simulink model "All_64_Coils"  (R2021b)
settings: Solver=FixedStepAuto  StartTime=-3000e-6  StopTime=3000e-6  FixedStep=25e-6  RelTol=1e-3
(part 1 of 70)
settings Model: Creator=teja  LastModifiedBy=teja

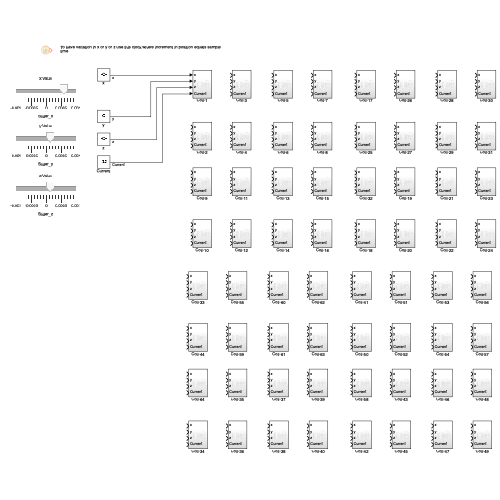
[thumbnail: Simulink's stored preview of the top-level diagram]
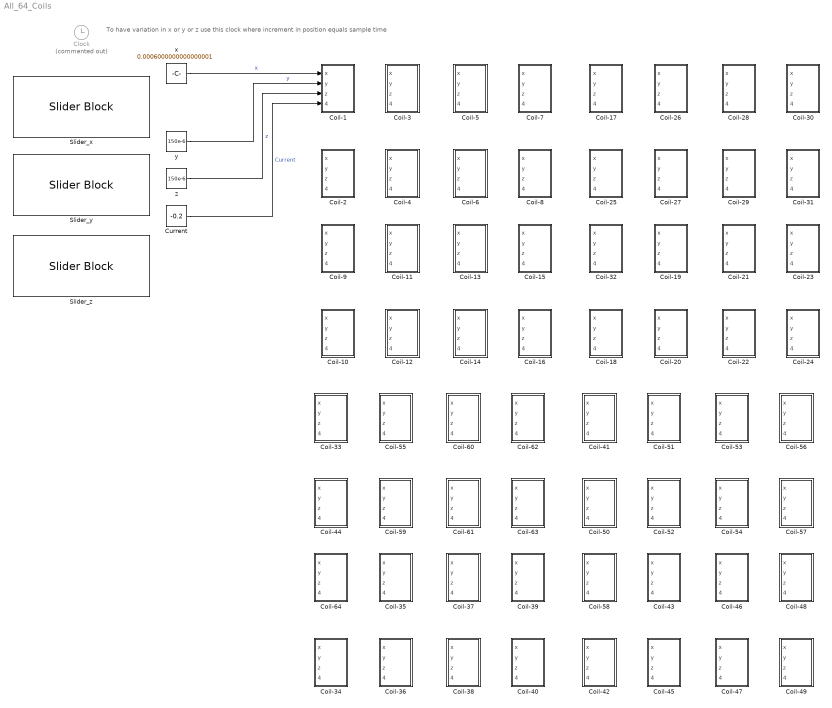
== system All_64_Coils ==
  note: To have variation in x or y or z use this clock where increment in position equals sample time
  Clock : Clock  Commented=on
  Coil-1 : Subsystem
  Coil-10 : Subsystem
  Coil-11 : Subsystem
  Coil-12 : Subsystem
  Coil-13 : Subsystem
  Coil-14 : Subsystem
  Coil-15 : Subsystem
  Coil-16 : Subsystem
  Coil-17 : Subsystem
  Coil-18 : Subsystem
  Coil-19 : Subsystem
  Coil-2 : Subsystem
  Coil-20 : Subsystem
  Coil-21 : Subsystem
  Coil-22 : Subsystem
  Coil-23 : Subsystem
  Coil-24 : Subsystem
  Coil-25 : Subsystem
  Coil-26 : Subsystem
  Coil-27 : Subsystem
  Coil-28 : Subsystem
  Coil-29 : Subsystem
  Coil-3 : Subsystem
  Coil-30 : Subsystem
  Coil-31 : Subsystem
  Coil-32 : Subsystem
  Coil-33 : Subsystem
  Coil-34 : Subsystem
  Coil-35 : Subsystem
  Coil-36 : Subsystem
  Coil-37 : Subsystem
  Coil-38 : Subsystem
  Coil-39 : Subsystem
  Coil-4 : Subsystem
  Coil-40 : Subsystem
  Coil-41 : Subsystem
  Coil-42 : Subsystem
  Coil-43 : Subsystem
  Coil-44 : Subsystem
  Coil-45 : Subsystem
  Coil-46 : Subsystem
  Coil-47 : Subsystem
  Coil-48 : Subsystem
  Coil-49 : Subsystem
  Coil-5 : Subsystem
  Coil-50 : Subsystem
  Coil-51 : Subsystem
  Coil-52 : Subsystem
  Coil-53 : Subsystem
  Coil-54 : Subsystem
  Coil-55 : Subsystem
  Coil-56 : Subsystem
  Coil-57 : Subsystem
  Coil-58 : Subsystem
  Coil-59 : Subsystem
  Coil-6 : Subsystem
  Coil-60 : Subsystem
  Coil-61 : Subsystem
  Coil-62 : Subsystem
  Coil-63 : Subsystem
  Coil-64 : Subsystem
  Coil-7 : Subsystem
  Coil-8 : Subsystem
  Coil-9 : Subsystem
  Current : Constant  Value=-0.2
  Slider_x : SliderBlock  ScaleMin=-1000e-6  ScaleMax=1000e-6
  Slider_y : SliderBlock  ScaleMin=-1e-3  ScaleMax=1e-3
  Slider_z : SliderBlock  ScaleMin=-1000e-6  ScaleMax=1000e-6
  x : Constant  Value=0.0006000000000000001
  y : Constant  Value=150e-6
  z : Constant  Value=150e-6
  wires:
  x/1 -> Coil-1/1(x)  "x"
  y/1 -> Coil-1/2(y)  "y"
  z/1 -> Coil-1/3(z)  "z"
  Current/1 -> Coil-1/4(Current)  "Current"

== system All_64_Coils/Coil-1 ==
  x : Inport
  y : Inport  Port=2
  z : Inport  Port=3
  Current : Inport  Port=4
  Add{,1..4} (5 alike) : Sum
  Display{,1..3} (4 alike) : Display
  Inner_5_coils : Subsystem
  Outer_5_Coils : Subsystem
  Scope : Scope  NumInputPorts=4
  Scope1 : Scope
  wires:
  Add4/1 -> Scope1/1  "Total_Force"
  Inner_5_coils/3(Output2) -> Add2/1
  Current/1 -> Inner_5_coils/4(Input3), Outer_5_Coils/4(Input3)
  Outer_5_Coils/2(Output1) -> Add1/2
  Add2/1 -> Display2/1, Scope/3  "Fyy"
  x/1 -> Inner_5_coils/1(Input), Outer_5_Coils/1(Input)
  Outer_5_Coils/1(Output) -> Add/2
  Add1/1 -> Add4/1, Display1/1, Scope/2  "Fxz"
  Add3/1 -> Add4/2, Display3/1, Scope/4  "Fyz"
  Inner_5_coils/1(Output) -> Add/1
  Inner_5_coils/4(Output3) -> Add3/1
  y/1 -> Inner_5_coils/2(Input1), Outer_5_Coils/2(Input1)
  Add/1 -> Display/1, Scope/1  "Fxx"
  z/1 -> Inner_5_coils/3(Input2), Outer_5_Coils/3(Input2)
  Outer_5_Coils/4(Output3) -> Add3/2
  Inner_5_coils/2(Output1) -> Add1/1
  Outer_5_Coils/3(Output2) -> Add2/2

== system All_64_Coils/Coil-10 ==
  x : Inport
  y : Inport  Port=2
  z : Inport  Port=3
  Current : Inport  Port=4
  Add{,1..4} (5 alike) : Sum
  Display{,1..3} (4 alike) : Display
  Inner_5_coils : Subsystem
  Outer_5_Coils : Subsystem
  Scope : Scope  NumInputPorts=4
  Scope1 : Scope
  wires:
  Add4/1 -> Scope1/1  "Total_Force"
  Inner_5_coils/3(Output2) -> Add2/1
  Current/1 -> Inner_5_coils/4(Input3), Outer_5_Coils/4(Input3)
  Outer_5_Coils/2(Output1) -> Add1/2
  Add2/1 -> Display2/1, Scope/3  "Fyy"
  x/1 -> Inner_5_coils/1(Input), Outer_5_Coils/1(Input)
  Outer_5_Coils/1(Output) -> Add/2
  Add1/1 -> Add4/1, Display1/1, Scope/2  "Fxz"
  Add3/1 -> Add4/2, Display3/1, Scope/4  "Fyz"
  Inner_5_coils/1(Output) -> Add/1
  Inner_5_coils/4(Output3) -> Add3/1
  y/1 -> Inner_5_coils/2(Input1), Outer_5_Coils/2(Input1)
  Add/1 -> Display/1, Scope/1  "Fxx"
  z/1 -> Inner_5_coils/3(Input2), Outer_5_Coils/3(Input2)
  Outer_5_Coils/4(Output3) -> Add3/2
  Inner_5_coils/2(Output1) -> Add1/1
  Outer_5_Coils/3(Output2) -> Add2/2

== system All_64_Coils/Coil-11 ==
  x : Inport
  y : Inport  Port=2
  z : Inport  Port=3
  Current : Inport  Port=4
  Add{,1..4} (5 alike) : Sum
  Display{,1..3} (4 alike) : Display
  Inner_5_coils : Subsystem
  Outer_5_Coils : Subsystem
  Scope : Scope  NumInputPorts=4
  Scope1 : Scope
  wires:
  Add4/1 -> Scope1/1  "Total_Force"
  Inner_5_coils/3(Output2) -> Add2/1
  Current/1 -> Inner_5_coils/4(Input3), Outer_5_Coils/4(Input3)
  Outer_5_Coils/2(Output1) -> Add1/2
  Add2/1 -> Display2/1, Scope/3  "Fyy"
  x/1 -> Inner_5_coils/1(Input), Outer_5_Coils/1(Input)
  Outer_5_Coils/1(Output) -> Add/2
  Add1/1 -> Add4/1, Display1/1, Scope/2  "Fxz"
  Add3/1 -> Add4/2, Display3/1, Scope/4  "Fyz"
  Inner_5_coils/1(Output) -> Add/1
  Inner_5_coils/4(Output3) -> Add3/1
  y/1 -> Inner_5_coils/2(Input1), Outer_5_Coils/2(Input1)
  Add/1 -> Display/1, Scope/1  "Fxx"
  z/1 -> Inner_5_coils/3(Input2), Outer_5_Coils/3(Input2)
  Outer_5_Coils/4(Output3) -> Add3/2
  Inner_5_coils/2(Output1) -> Add1/1
  Outer_5_Coils/3(Output2) -> Add2/2

== system All_64_Coils/Coil-12 ==
  x : Inport
  y : Inport  Port=2
  z : Inport  Port=3
  Current : Inport  Port=4
  Add{,1..4} (5 alike) : Sum
  Display{,1..3} (4 alike) : Display
  Inner_5_coils : Subsystem
  Outer_5_Coils : Subsystem
  Scope : Scope  NumInputPorts=4
  Scope1 : Scope
  wires:
  Outer_5_Coils/3(Output2) -> Add2/2
  Inner_5_coils/2(Output1) -> Add1/1
  Outer_5_Coils/4(Output3) -> Add3/2
  z/1 -> Outer_5_Coils/3(Input2), Inner_5_coils/3(Input2)
  Add/1 -> Scope/1, Display/1  "Fxx"
  y/1 -> Outer_5_Coils/2(Input1), Inner_5_coils/2(Input1)
  Inner_5_coils/4(Output3) -> Add3/1
  Inner_5_coils/1(Output) -> Add/1
  Add3/1 -> Scope/4, Display3/1, Add4/2  "Fyz"
  Add1/1 -> Scope/2, Display1/1, Add4/1  "Fxz"
  Outer_5_Coils/1(Output) -> Add/2
  x/1 -> Outer_5_Coils/1(Input), Inner_5_coils/1(Input)
  Add2/1 -> Scope/3, Display2/1  "Fyy"
  Outer_5_Coils/2(Output1) -> Add1/2
  Current/1 -> Outer_5_Coils/4(Input3), Inner_5_coils/4(Input3)
  Inner_5_coils/3(Output2) -> Add2/1
  Add4/1 -> Scope1/1  "Total_Force"

== system All_64_Coils/Coil-13 ==
  x : Inport
  y : Inport  Port=2
  z : Inport  Port=3
  Current : Inport  Port=4
  Add{,1..4} (5 alike) : Sum
  Display{,1..3} (4 alike) : Display
  Inner_5_coils : Subsystem
  Outer_5_Coils : Subsystem
  Scope : Scope  NumInputPorts=4
  Scope1 : Scope
  wires:
  Add4/1 -> Scope1/1  "Total_Force"
  Inner_5_coils/3(Output2) -> Add2/1
  Current/1 -> Inner_5_coils/4(Input3), Outer_5_Coils/4(Input3)
  Outer_5_Coils/2(Output1) -> Add1/2
  Add2/1 -> Display2/1, Scope/3  "Fyy"
  x/1 -> Inner_5_coils/1(Input), Outer_5_Coils/1(Input)
  Outer_5_Coils/1(Output) -> Add/2
  Add1/1 -> Add4/1, Display1/1, Scope/2  "Fxz"
  Add3/1 -> Add4/2, Display3/1, Scope/4  "Fyz"
  Inner_5_coils/1(Output) -> Add/1
  Inner_5_coils/4(Output3) -> Add3/1
  y/1 -> Inner_5_coils/2(Input1), Outer_5_Coils/2(Input1)
  Add/1 -> Display/1, Scope/1  "Fxx"
  z/1 -> Inner_5_coils/3(Input2), Outer_5_Coils/3(Input2)
  Outer_5_Coils/4(Output3) -> Add3/2
  Inner_5_coils/2(Output1) -> Add1/1
  Outer_5_Coils/3(Output2) -> Add2/2

== system All_64_Coils/Coil-14 ==
  x : Inport
  y : Inport  Port=2
  z : Inport  Port=3
  Current : Inport  Port=4
  Add{,1..4} (5 alike) : Sum
  Display{,1..3} (4 alike) : Display
  Inner_5_coils : Subsystem
  Outer_5_Coils : Subsystem
  Scope : Scope  NumInputPorts=4
  Scope1 : Scope
  wires:
  Outer_5_Coils/3(Output2) -> Add2/2
  Inner_5_coils/2(Output1) -> Add1/1
  Outer_5_Coils/4(Output3) -> Add3/2
  z/1 -> Outer_5_Coils/3(Input2), Inner_5_coils/3(Input2)
  Add/1 -> Scope/1, Display/1  "Fxx"
  y/1 -> Outer_5_Coils/2(Input1), Inner_5_coils/2(Input1)
  Inner_5_coils/4(Output3) -> Add3/1
  Inner_5_coils/1(Output) -> Add/1
  Add3/1 -> Scope/4, Display3/1, Add4/2  "Fyz"
  Add1/1 -> Scope/2, Display1/1, Add4/1  "Fxz"
  Outer_5_Coils/1(Output) -> Add/2
  x/1 -> Outer_5_Coils/1(Input), Inner_5_coils/1(Input)
  Add2/1 -> Scope/3, Display2/1  "Fyy"
  Outer_5_Coils/2(Output1) -> Add1/2
  Current/1 -> Outer_5_Coils/4(Input3), Inner_5_coils/4(Input3)
  Inner_5_coils/3(Output2) -> Add2/1
  Add4/1 -> Scope1/1  "Total_Force"

== system All_64_Coils/Coil-15 ==
  x : Inport
  y : Inport  Port=2
  z : Inport  Port=3
  Current : Inport  Port=4
  Add{,1..4} (5 alike) : Sum
  Display{,1..3} (4 alike) : Display
  Inner_5_coils : Subsystem
  Outer_5_Coils : Subsystem
  Scope : Scope  NumInputPorts=4
  Scope1 : Scope
  wires:
  Outer_5_Coils/3(Output2) -> Add2/2
  Inner_5_coils/2(Output1) -> Add1/1
  Outer_5_Coils/4(Output3) -> Add3/2
  z/1 -> Outer_5_Coils/3(Input2), Inner_5_coils/3(Input2)
  Add/1 -> Scope/1, Display/1  "Fxx"
  y/1 -> Outer_5_Coils/2(Input1), Inner_5_coils/2(Input1)
  Inner_5_coils/4(Output3) -> Add3/1
  Inner_5_coils/1(Output) -> Add/1
  Add3/1 -> Scope/4, Display3/1, Add4/2  "Fyz"
  Add1/1 -> Scope/2, Display1/1, Add4/1  "Fxz"
  Outer_5_Coils/1(Output) -> Add/2
  x/1 -> Outer_5_Coils/1(Input), Inner_5_coils/1(Input)
  Add2/1 -> Scope/3, Display2/1  "Fyy"
  Outer_5_Coils/2(Output1) -> Add1/2
  Current/1 -> Outer_5_Coils/4(Input3), Inner_5_coils/4(Input3)
  Inner_5_coils/3(Output2) -> Add2/1
  Add4/1 -> Scope1/1  "Total_Force"

== system All_64_Coils/Coil-16 ==
  x : Inport
  y : Inport  Port=2
  z : Inport  Port=3
  Current : Inport  Port=4
  Add{,1..4} (5 alike) : Sum
  Display{,1..3} (4 alike) : Display
  Inner_5_coils : Subsystem
  Outer_5_Coils : Subsystem
  Scope : Scope  NumInputPorts=4
  Scope1 : Scope
  wires:
  Add4/1 -> Scope1/1  "Total_Force"
  Inner_5_coils/3(Output2) -> Add2/1
  Current/1 -> Inner_5_coils/4(Input3), Outer_5_Coils/4(Input3)
  Outer_5_Coils/2(Output1) -> Add1/2
  Add2/1 -> Display2/1, Scope/3  "Fyy"
  x/1 -> Inner_5_coils/1(Input), Outer_5_Coils/1(Input)
  Outer_5_Coils/1(Output) -> Add/2
  Add1/1 -> Add4/1, Display1/1, Scope/2  "Fxz"
  Add3/1 -> Add4/2, Display3/1, Scope/4  "Fyz"
  Inner_5_coils/1(Output) -> Add/1
  Inner_5_coils/4(Output3) -> Add3/1
  y/1 -> Inner_5_coils/2(Input1), Outer_5_Coils/2(Input1)
  Add/1 -> Display/1, Scope/1  "Fxx"
  z/1 -> Inner_5_coils/3(Input2), Outer_5_Coils/3(Input2)
  Outer_5_Coils/4(Output3) -> Add3/2
  Inner_5_coils/2(Output1) -> Add1/1
  Outer_5_Coils/3(Output2) -> Add2/2

== system All_64_Coils/Coil-17 ==
  x : Inport
  y : Inport  Port=2
  z : Inport  Port=3
  Current : Inport  Port=4
  Add{,1..4} (5 alike) : Sum
  Display{,1..3} (4 alike) : Display
  Inner_5_coils : Subsystem
  Outer_5_Coils : Subsystem
  Scope : Scope  NumInputPorts=4
  Scope1 : Scope
  wires:
  Outer_5_Coils/3(Output2) -> Add2/2
  Inner_5_coils/2(Output1) -> Add1/1
  Outer_5_Coils/4(Output3) -> Add3/2
  z/1 -> Outer_5_Coils/3(Input2), Inner_5_coils/3(Input2)
  Add/1 -> Scope/1, Display/1  "Fxx"
  y/1 -> Outer_5_Coils/2(Input1), Inner_5_coils/2(Input1)
  Inner_5_coils/4(Output3) -> Add3/1
  Inner_5_coils/1(Output) -> Add/1
  Add3/1 -> Scope/4, Display3/1, Add4/2  "Fyz"
  Add1/1 -> Scope/2, Display1/1, Add4/1  "Fxz"
  Outer_5_Coils/1(Output) -> Add/2
  x/1 -> Outer_5_Coils/1(Input), Inner_5_coils/1(Input)
  Add2/1 -> Scope/3, Display2/1  "Fyy"
  Outer_5_Coils/2(Output1) -> Add1/2
  Current/1 -> Outer_5_Coils/4(Input3), Inner_5_coils/4(Input3)
  Inner_5_coils/3(Output2) -> Add2/1
  Add4/1 -> Scope1/1  "Total_Force"

== system All_64_Coils/Coil-18 ==
  x : Inport
  y : Inport  Port=2
  z : Inport  Port=3
  Current : Inport  Port=4
  Add{,1..4} (5 alike) : Sum
  Display{,1..3} (4 alike) : Display
  Inner_5_coils : Subsystem
  Outer_5_Coils : Subsystem
  Scope : Scope  NumInputPorts=4
  Scope1 : Scope
  wires:
  Outer_5_Coils/3(Output2) -> Add2/2
  Inner_5_coils/2(Output1) -> Add1/1
  Outer_5_Coils/4(Output3) -> Add3/2
  z/1 -> Outer_5_Coils/3(Input2), Inner_5_coils/3(Input2)
  Add/1 -> Scope/1, Display/1  "Fxx"
  y/1 -> Outer_5_Coils/2(Input1), Inner_5_coils/2(Input1)
  Inner_5_coils/4(Output3) -> Add3/1
  Inner_5_coils/1(Output) -> Add/1
  Add3/1 -> Scope/4, Display3/1, Add4/2  "Fyz"
  Add1/1 -> Scope/2, Display1/1, Add4/1  "Fxz"
  Outer_5_Coils/1(Output) -> Add/2
  x/1 -> Outer_5_Coils/1(Input), Inner_5_coils/1(Input)
  Add2/1 -> Scope/3, Display2/1  "Fyy"
  Outer_5_Coils/2(Output1) -> Add1/2
  Current/1 -> Outer_5_Coils/4(Input3), Inner_5_coils/4(Input3)
  Inner_5_coils/3(Output2) -> Add2/1
  Add4/1 -> Scope1/1  "Total_Force"

== system All_64_Coils/Coil-19 ==
  x : Inport
  y : Inport  Port=2
  z : Inport  Port=3
  Current : Inport  Port=4
  Add{,1..4} (5 alike) : Sum
  Display{,1..3} (4 alike) : Display
  Inner_5_coils : Subsystem
  Outer_5_Coils : Subsystem
  Scope : Scope  NumInputPorts=4
  Scope1 : Scope
  wires:
  Outer_5_Coils/3(Output2) -> Add2/2
  Inner_5_coils/2(Output1) -> Add1/1
  Outer_5_Coils/4(Output3) -> Add3/2
  z/1 -> Outer_5_Coils/3(Input2), Inner_5_coils/3(Input2)
  Add/1 -> Scope/1, Display/1  "Fxx"
  y/1 -> Outer_5_Coils/2(Input1), Inner_5_coils/2(Input1)
  Inner_5_coils/4(Output3) -> Add3/1
  Inner_5_coils/1(Output) -> Add/1
  Add3/1 -> Scope/4, Display3/1, Add4/2  "Fyz"
  Add1/1 -> Scope/2, Display1/1, Add4/1  "Fxz"
  Outer_5_Coils/1(Output) -> Add/2
  x/1 -> Outer_5_Coils/1(Input), Inner_5_coils/1(Input)
  Add2/1 -> Scope/3, Display2/1  "Fyy"
  Outer_5_Coils/2(Output1) -> Add1/2
  Current/1 -> Outer_5_Coils/4(Input3), Inner_5_coils/4(Input3)
  Inner_5_coils/3(Output2) -> Add2/1
  Add4/1 -> Scope1/1  "Total_Force"

== system All_64_Coils/Coil-2 ==
  x : Inport
  y : Inport  Port=2
  z : Inport  Port=3
  Current : Inport  Port=4
  Add{,1..4} (5 alike) : Sum
  Display{,1..3} (4 alike) : Display
  Inner_5_coils : Subsystem
  Outer_5_Coils : Subsystem
  Scope : Scope  NumInputPorts=4
  Scope1 : Scope
  wires:
  Outer_5_Coils/3(Output2) -> Add2/2
  Inner_5_coils/2(Output1) -> Add1/1
  Outer_5_Coils/4(Output3) -> Add3/2
  z/1 -> Outer_5_Coils/3(Input2), Inner_5_coils/3(Input2)
  Add/1 -> Scope/1, Display/1  "Fxx"
  y/1 -> Outer_5_Coils/2(Input1), Inner_5_coils/2(Input1)
  Inner_5_coils/4(Output3) -> Add3/1
  Inner_5_coils/1(Output) -> Add/1
  Add3/1 -> Scope/4, Display3/1, Add4/2  "Fyz"
  Add1/1 -> Scope/2, Display1/1, Add4/1  "Fxz"
  Outer_5_Coils/1(Output) -> Add/2
  x/1 -> Outer_5_Coils/1(Input), Inner_5_coils/1(Input)
  Add2/1 -> Scope/3, Display2/1  "Fyy"
  Outer_5_Coils/2(Output1) -> Add1/2
  Current/1 -> Outer_5_Coils/4(Input3), Inner_5_coils/4(Input3)
  Inner_5_coils/3(Output2) -> Add2/1
  Add4/1 -> Scope1/1  "Total_Force"

== system All_64_Coils/Coil-20 ==
  x : Inport
  y : Inport  Port=2
  z : Inport  Port=3
  Current : Inport  Port=4
  Add{,1..4} (5 alike) : Sum
  Display{,1..3} (4 alike) : Display
  Inner_5_coils : Subsystem
  Outer_5_Coils : Subsystem
  Scope : Scope  NumInputPorts=4
  Scope1 : Scope
  wires:
  Add4/1 -> Scope1/1  "Total_Force"
  Inner_5_coils/3(Output2) -> Add2/1
  Current/1 -> Inner_5_coils/4(Input3), Outer_5_Coils/4(Input3)
  Outer_5_Coils/2(Output1) -> Add1/2
  Add2/1 -> Display2/1, Scope/3  "Fyy"
  x/1 -> Inner_5_coils/1(Input), Outer_5_Coils/1(Input)
  Outer_5_Coils/1(Output) -> Add/2
  Add1/1 -> Add4/1, Display1/1, Scope/2  "Fxz"
  Add3/1 -> Add4/2, Display3/1, Scope/4  "Fyz"
  Inner_5_coils/1(Output) -> Add/1
  Inner_5_coils/4(Output3) -> Add3/1
  y/1 -> Inner_5_coils/2(Input1), Outer_5_Coils/2(Input1)
  Add/1 -> Display/1, Scope/1  "Fxx"
  z/1 -> Inner_5_coils/3(Input2), Outer_5_Coils/3(Input2)
  Outer_5_Coils/4(Output3) -> Add3/2
  Inner_5_coils/2(Output1) -> Add1/1
  Outer_5_Coils/3(Output2) -> Add2/2

== system All_64_Coils/Coil-21 ==
  x : Inport
  y : Inport  Port=2
  z : Inport  Port=3
  Current : Inport  Port=4
  Add{,1..4} (5 alike) : Sum
  Display{,1..3} (4 alike) : Display
  Inner_5_coils : Subsystem
  Outer_5_Coils : Subsystem
  Scope : Scope  NumInputPorts=4
  Scope1 : Scope
  wires:
  Outer_5_Coils/3(Output2) -> Add2/2
  Inner_5_coils/2(Output1) -> Add1/1
  Outer_5_Coils/4(Output3) -> Add3/2
  z/1 -> Outer_5_Coils/3(Input2), Inner_5_coils/3(Input2)
  Add/1 -> Scope/1, Display/1  "Fxx"
  y/1 -> Outer_5_Coils/2(Input1), Inner_5_coils/2(Input1)
  Inner_5_coils/4(Output3) -> Add3/1
  Inner_5_coils/1(Output) -> Add/1
  Add3/1 -> Scope/4, Display3/1, Add4/2  "Fyz"
  Add1/1 -> Scope/2, Display1/1, Add4/1  "Fxz"
  Outer_5_Coils/1(Output) -> Add/2
  x/1 -> Outer_5_Coils/1(Input), Inner_5_coils/1(Input)
  Add2/1 -> Scope/3, Display2/1  "Fyy"
  Outer_5_Coils/2(Output1) -> Add1/2
  Current/1 -> Outer_5_Coils/4(Input3), Inner_5_coils/4(Input3)
  Inner_5_coils/3(Output2) -> Add2/1
  Add4/1 -> Scope1/1  "Total_Force"

== system All_64_Coils/Coil-22 ==
  x : Inport
  y : Inport  Port=2
  z : Inport  Port=3
  Current : Inport  Port=4
  Add{,1..4} (5 alike) : Sum
  Display{,1..3} (4 alike) : Display
  Inner_5_coils : Subsystem
  Outer_5_Coils : Subsystem
  Scope : Scope  NumInputPorts=4
  Scope1 : Scope
  wires:
  Add4/1 -> Scope1/1  "Total_Force"
  Inner_5_coils/3(Output2) -> Add2/1
  Current/1 -> Inner_5_coils/4(Input3), Outer_5_Coils/4(Input3)
  Outer_5_Coils/2(Output1) -> Add1/2
  Add2/1 -> Display2/1, Scope/3  "Fyy"
  x/1 -> Inner_5_coils/1(Input), Outer_5_Coils/1(Input)
  Outer_5_Coils/1(Output) -> Add/2
  Add1/1 -> Add4/1, Display1/1, Scope/2  "Fxz"
  Add3/1 -> Add4/2, Display3/1, Scope/4  "Fyz"
  Inner_5_coils/1(Output) -> Add/1
  Inner_5_coils/4(Output3) -> Add3/1
  y/1 -> Inner_5_coils/2(Input1), Outer_5_Coils/2(Input1)
  Add/1 -> Display/1, Scope/1  "Fxx"
  z/1 -> Inner_5_coils/3(Input2), Outer_5_Coils/3(Input2)
  Outer_5_Coils/4(Output3) -> Add3/2
  Inner_5_coils/2(Output1) -> Add1/1
  Outer_5_Coils/3(Output2) -> Add2/2

== system All_64_Coils/Coil-23 ==
  x : Inport
  y : Inport  Port=2
  z : Inport  Port=3
  Current : Inport  Port=4
  Add{,1..4} (5 alike) : Sum
  Display{,1..3} (4 alike) : Display
  Inner_5_coils : Subsystem
  Outer_5_Coils : Subsystem
  Scope : Scope  NumInputPorts=4
  Scope1 : Scope
  wires:
  Add4/1 -> Scope1/1  "Total_Force"
  Inner_5_coils/3(Output2) -> Add2/1
  Current/1 -> Inner_5_coils/4(Input3), Outer_5_Coils/4(Input3)
  Outer_5_Coils/2(Output1) -> Add1/2
  Add2/1 -> Display2/1, Scope/3  "Fyy"
  x/1 -> Inner_5_coils/1(Input), Outer_5_Coils/1(Input)
  Outer_5_Coils/1(Output) -> Add/2
  Add1/1 -> Add4/1, Display1/1, Scope/2  "Fxz"
  Add3/1 -> Add4/2, Display3/1, Scope/4  "Fyz"
  Inner_5_coils/1(Output) -> Add/1
  Inner_5_coils/4(Output3) -> Add3/1
  y/1 -> Inner_5_coils/2(Input1), Outer_5_Coils/2(Input1)
  Add/1 -> Display/1, Scope/1  "Fxx"
  z/1 -> Inner_5_coils/3(Input2), Outer_5_Coils/3(Input2)
  Outer_5_Coils/4(Output3) -> Add3/2
  Inner_5_coils/2(Output1) -> Add1/1
  Outer_5_Coils/3(Output2) -> Add2/2

== system All_64_Coils/Coil-24 ==
  x : Inport
  y : Inport  Port=2
  z : Inport  Port=3
  Current : Inport  Port=4
  Add{,1..4} (5 alike) : Sum
  Display{,1..3} (4 alike) : Display
  Inner_5_coils : Subsystem
  Outer_5_Coils : Subsystem
  Scope : Scope  NumInputPorts=4
  Scope1 : Scope
  wires:
  Outer_5_Coils/3(Output2) -> Add2/2
  Inner_5_coils/2(Output1) -> Add1/1
  Outer_5_Coils/4(Output3) -> Add3/2
  z/1 -> Outer_5_Coils/3(Input2), Inner_5_coils/3(Input2)
  Add/1 -> Scope/1, Display/1  "Fxx"
  y/1 -> Outer_5_Coils/2(Input1), Inner_5_coils/2(Input1)
  Inner_5_coils/4(Output3) -> Add3/1
  Inner_5_coils/1(Output) -> Add/1
  Add3/1 -> Scope/4, Display3/1, Add4/2  "Fyz"
  Add1/1 -> Scope/2, Display1/1, Add4/1  "Fxz"
  Outer_5_Coils/1(Output) -> Add/2
  x/1 -> Outer_5_Coils/1(Input), Inner_5_coils/1(Input)
  Add2/1 -> Scope/3, Display2/1  "Fyy"
  Outer_5_Coils/2(Output1) -> Add1/2
  Current/1 -> Outer_5_Coils/4(Input3), Inner_5_coils/4(Input3)
  Inner_5_coils/3(Output2) -> Add2/1
  Add4/1 -> Scope1/1  "Total_Force"

== system All_64_Coils/Coil-25 ==
  x : Inport
  y : Inport  Port=2
  z : Inport  Port=3
  Current : Inport  Port=4
  Add{,1..4} (5 alike) : Sum
  Display{,1..3} (4 alike) : Display
  Inner_5_coils : Subsystem
  Outer_5_Coils : Subsystem
  Scope : Scope  NumInputPorts=4
  Scope1 : Scope
  wires:
  Add4/1 -> Scope1/1  "Total_Force"
  Inner_5_coils/3(Output2) -> Add2/1
  Current/1 -> Inner_5_coils/4(Input3), Outer_5_Coils/4(Input3)
  Outer_5_Coils/2(Output1) -> Add1/2
  Add2/1 -> Display2/1, Scope/3  "Fyy"
  x/1 -> Inner_5_coils/1(Input), Outer_5_Coils/1(Input)
  Outer_5_Coils/1(Output) -> Add/2
  Add1/1 -> Add4/1, Display1/1, Scope/2  "Fxz"
  Add3/1 -> Add4/2, Display3/1, Scope/4  "Fyz"
  Inner_5_coils/1(Output) -> Add/1
  Inner_5_coils/4(Output3) -> Add3/1
  y/1 -> Inner_5_coils/2(Input1), Outer_5_Coils/2(Input1)
  Add/1 -> Display/1, Scope/1  "Fxx"
  z/1 -> Inner_5_coils/3(Input2), Outer_5_Coils/3(Input2)
  Outer_5_Coils/4(Output3) -> Add3/2
  Inner_5_coils/2(Output1) -> Add1/1
  Outer_5_Coils/3(Output2) -> Add2/2

== system All_64_Coils/Coil-26 ==
  x : Inport
  y : Inport  Port=2
  z : Inport  Port=3
  Current : Inport  Port=4
  Add{,1..4} (5 alike) : Sum
  Display{,1..3} (4 alike) : Display
  Inner_5_coils : Subsystem
  Outer_5_Coils : Subsystem
  Scope : Scope  NumInputPorts=4
  Scope1 : Scope
  wires:
  Add4/1 -> Scope1/1  "Total_Force"
  Inner_5_coils/3(Output2) -> Add2/1
  Current/1 -> Inner_5_coils/4(Input3), Outer_5_Coils/4(Input3)
  Outer_5_Coils/2(Output1) -> Add1/2
  Add2/1 -> Display2/1, Scope/3  "Fyy"
  x/1 -> Inner_5_coils/1(Input), Outer_5_Coils/1(Input)
  Outer_5_Coils/1(Output) -> Add/2
  Add1/1 -> Add4/1, Display1/1, Scope/2  "Fxz"
  Add3/1 -> Add4/2, Display3/1, Scope/4  "Fyz"
  Inner_5_coils/1(Output) -> Add/1
  Inner_5_coils/4(Output3) -> Add3/1
  y/1 -> Inner_5_coils/2(Input1), Outer_5_Coils/2(Input1)
  Add/1 -> Display/1, Scope/1  "Fxx"
  z/1 -> Inner_5_coils/3(Input2), Outer_5_Coils/3(Input2)
  Outer_5_Coils/4(Output3) -> Add3/2
  Inner_5_coils/2(Output1) -> Add1/1
  Outer_5_Coils/3(Output2) -> Add2/2

== system All_64_Coils/Coil-27 ==
  x : Inport
  y : Inport  Port=2
  z : Inport  Port=3
  Current : Inport  Port=4
  Add{,1..4} (5 alike) : Sum
  Display{,1..3} (4 alike) : Display
  Inner_5_coils : Subsystem
  Outer_5_Coils : Subsystem
  Scope : Scope  NumInputPorts=4
  Scope1 : Scope
  wires:
  Outer_5_Coils/3(Output2) -> Add2/2
  Inner_5_coils/2(Output1) -> Add1/1
  Outer_5_Coils/4(Output3) -> Add3/2
  z/1 -> Outer_5_Coils/3(Input2), Inner_5_coils/3(Input2)
  Add/1 -> Scope/1, Display/1  "Fxx"
  y/1 -> Outer_5_Coils/2(Input1), Inner_5_coils/2(Input1)
  Inner_5_coils/4(Output3) -> Add3/1
  Inner_5_coils/1(Output) -> Add/1
  Add3/1 -> Scope/4, Display3/1, Add4/2  "Fyz"
  Add1/1 -> Scope/2, Display1/1, Add4/1  "Fxz"
  Outer_5_Coils/1(Output) -> Add/2
  x/1 -> Outer_5_Coils/1(Input), Inner_5_coils/1(Input)
  Add2/1 -> Scope/3, Display2/1  "Fyy"
  Outer_5_Coils/2(Output1) -> Add1/2
  Current/1 -> Outer_5_Coils/4(Input3), Inner_5_coils/4(Input3)
  Inner_5_coils/3(Output2) -> Add2/1
  Add4/1 -> Scope1/1  "Total_Force"

== system All_64_Coils/Coil-28 ==
  x : Inport
  y : Inport  Port=2
  z : Inport  Port=3
  Current : Inport  Port=4
  Add{,1..4} (5 alike) : Sum
  Display{,1..3} (4 alike) : Display
  Inner_5_coils : Subsystem
  Outer_5_Coils : Subsystem
  Scope : Scope  NumInputPorts=4
  Scope1 : Scope
  wires:
  Add4/1 -> Scope1/1  "Total_Force"
  Inner_5_coils/3(Output2) -> Add2/1
  Current/1 -> Inner_5_coils/4(Input3), Outer_5_Coils/4(Input3)
  Outer_5_Coils/2(Output1) -> Add1/2
  Add2/1 -> Display2/1, Scope/3  "Fyy"
  x/1 -> Inner_5_coils/1(Input), Outer_5_Coils/1(Input)
  Outer_5_Coils/1(Output) -> Add/2
  Add1/1 -> Add4/1, Display1/1, Scope/2  "Fxz"
  Add3/1 -> Add4/2, Display3/1, Scope/4  "Fyz"
  Inner_5_coils/1(Output) -> Add/1
  Inner_5_coils/4(Output3) -> Add3/1
  y/1 -> Inner_5_coils/2(Input1), Outer_5_Coils/2(Input1)
  Add/1 -> Display/1, Scope/1  "Fxx"
  z/1 -> Inner_5_coils/3(Input2), Outer_5_Coils/3(Input2)
  Outer_5_Coils/4(Output3) -> Add3/2
  Inner_5_coils/2(Output1) -> Add1/1
  Outer_5_Coils/3(Output2) -> Add2/2
